simulink library "lib_all"  (Simulink 7.6)
settings: Solver=ode45  SolverMode=SingleTasking  StartTime=0.0  StopTime=10.0  RelTol=1e-3
values from neighbouring files (symbols this model uses):
  map_lib_03 = [6.8679 7.0159 7.4571 8.1849 9.1892 10.456 11.97 13.71 15.655 17.781 20.063 22.474 ... (803 elements, 73x11)]    [scr_simdata.mat]    (also in scr_simdata_all.mat)
  map_lib_06 = [2.5784 2.6727 2.9588 3.4453 4.1444 5.068 6.226 7.6242 9.2634 11.139 13.24 15.552 ... (803 elements, 73x11)]    [scr_simdata.mat]    (also in scr_simdata_all.mat)
  map_lib_09 = [0.0025784 0.0026727 0.0029588 0.0034453 0.0041444 0.005068 0.006226 0.0076242 0.0092634 0.011139 0.01324 0.015552 ... (803 elements, 73x11)]    [scr_simdata.mat]    (also in scr_simdata_all.mat)
  map_lib_10 = [2.74 2.75 2.76 2.77 2.78 2.79 2.8 2.81 2.82 2.83 2.84 2.85 ... (81 elements, 1x81)]    [scr_simdata.mat]    (also in scr_simdata_all.mat)
  map_lib_11 = [1.1983 1.2027 1.207 1.2113 1.2156 1.2198 1.2241 1.2283 1.2326 1.2368 1.241 1.2451 ... (81 elements, 1x81)]    [scr_simdata.mat]    (also in scr_simdata_all.mat)
  map_lib_12 = [2.74 2.75 2.76 2.77 2.78 2.79 2.8 2.81 2.82 2.83 2.84 2.85 ... (81 elements, 1x81)]    [scr_simdata.mat]    (also in scr_simdata_all.mat)
  map_lib_13 = [1.74 1.75 1.76 1.77 1.78 1.79 1.8 1.81 1.82 1.83 1.84 1.85 ... (81 elements, 1x81)]    [scr_simdata.mat]    (also in scr_simdata_all.mat)
  map_lib_16 = [0.00048828125 1.6159 1.8962 2.0821 2.2251 2.3426 2.4433 2.5318 2.611 2.683 2.749 2.8101 ... (1419 elements, 129x11)]    [scr_simdata.mat]    (also in scr_simdata_all.mat)
  map_mr2_01 = [0 800 1600 2400 3200 4000]    [scr_simdata.mat]    (also in scr_simdata_all.mat)
  map_mr2_02 = [1.32 1.32 1.33 1.34 1.36 1.38]    [scr_simdata.mat]    (also in scr_simdata_all.mat)
  map_mr2_03 = [0 800 1600 2400 3200 4000]    [scr_simdata.mat]    (also in scr_simdata_all.mat)
  map_mr2_04 = [1.32 1.32 1.33 1.34 1.36 1.38]    [scr_simdata.mat]    (also in scr_simdata_all.mat)
  map_mr2_05 = [0 800 1600 2400 3200 4000]    [scr_simdata.mat]    (also in scr_simdata_all.mat)
  map_mr2_06 = [1.32 1.32 1.33 1.34 1.36 1.38]    [scr_simdata.mat]    (also in scr_simdata_all.mat)
  Parameter1 = 6    [scr_simdata.mat]    (also in scr_simdata_all.mat)
  Parameter101 = 30    [scr_simdata.mat]    (also in scr_simdata_all.mat)
  Parameter102 = 12    [scr_simdata.mat]    (also in scr_simdata_all.mat)
  Parameter103 = -240    [scr_simdata.mat]    (also in scr_simdata_all.mat)
  Parameter108 = 48    [scr_simdata.mat]    (also in scr_simdata_all.mat)
  Parameter120 = 16    [scr_simdata.mat]    (also in scr_simdata_all.mat)
  Parameter121 = 87    [scr_simdata.mat]    (also in scr_simdata_all.mat)
  Parameter123 = 78    [scr_simdata.mat]    (also in scr_simdata_all.mat)
  Parameter124 = 60    [scr_simdata.mat]    (also in scr_simdata_all.mat)
  Parameter125 = -480    [scr_simdata.mat]    (also in scr_simdata_all.mat)
  Parameter130 = 96    [scr_simdata.mat]    (also in scr_simdata_all.mat)
  Parameter131 = 3    [scr_simdata.mat]    (also in scr_simdata_all.mat)
  Parameter144 = 3    [scr_simdata.mat]    (also in scr_simdata_all.mat)
  Parameter18 = 128    [scr_simdata.mat]    (also in scr_simdata_all.mat)
  Parameter19 = 16    [scr_simdata.mat]    (also in scr_simdata_all.mat)
  Parameter2 = 12    [scr_simdata.mat]    (also in scr_simdata_all.mat)
  Parameter20 = 7    [scr_simdata.mat]    (also in scr_simdata_all.mat)
  Parameter21 = 4    [scr_simdata.mat]    (also in scr_simdata_all.mat)
  Parameter3 = 18    [scr_simdata.mat]    (also in scr_simdata_all.mat)
  Parameter32 = 1    [scr_simdata.mat]    (also in scr_simdata_all.mat)
  Parameter34 = 24    [scr_simdata.mat]    (also in scr_simdata_all.mat)
  Parameter4 = 138    [scr_simdata.mat]    (also in scr_simdata_all.mat)
  Parameter42 = 48    [scr_simdata.mat]    (also in scr_simdata_all.mat)
  Parameter5 = 135    [scr_simdata.mat]    (also in scr_simdata_all.mat)
  Parameter55 = 0    [scr_simdata.mat]    (also in scr_simdata_all.mat)
  Parameter56 = 0    [scr_simdata.mat]    (also in scr_simdata_all.mat)
  Parameter57 = 1    [scr_simdata.mat]    (also in scr_simdata_all.mat)
  Parameter58 = 1    [scr_simdata.mat]    (also in scr_simdata_all.mat)
  Parameter59 = 2    [scr_simdata.mat]    (also in scr_simdata_all.mat)
  Parameter6 = 132    [scr_simdata.mat]    (also in scr_simdata_all.mat)
  Parameter60 = 2    [scr_simdata.mat]    (also in scr_simdata_all.mat)
  Parameter63 = 144    [scr_simdata.mat]    (also in scr_simdata_all.mat)
  Parameter7 = 126    [scr_simdata.mat]    (also in scr_simdata_all.mat)
  Parameter76 = 64    [scr_simdata.mat]    (also in scr_simdata_all.mat)
  Parameter77 = 135    [scr_simdata.mat]    (also in scr_simdata_all.mat)
  Parameter79 = 126    [scr_simdata.mat]    (also in scr_simdata_all.mat)
  Parameter80 = 108    [scr_simdata.mat]    (also in scr_simdata_all.mat)
  Parameter81 = 0    [scr_simdata.mat]    (also in scr_simdata_all.mat)
  Parameter86 = 0    [scr_simdata.mat]    (also in scr_simdata_all.mat)
  Parameter98 = 112    [scr_simdata.mat]    (also in scr_simdata_all.mat)
  Parameter99 = 39    [scr_simdata.mat]    (also in scr_simdata_all.mat)
undefined symbols (not defined in the file or in its folder): cylvolkp_hih_map2h, cylvolkp_map2h, pk1k_map2h
libraries: simulink x2
(part 4 of 36)

== system lib_all/SubSystem1879/SubSystem1881 ==
  note: Text370
  note: Text369
  Block5146 : Inport
  Block5147 : Inport  Port=2
  Block5148 : Constant  Value=0  OutDataTypeStr=double  SampleTime=-1
  Block5149 : Constant  Value=0  OutDataTypeStr=double  SampleTime=-1
  Block5150 : Constant  Value=720  OutDataTypeStr=double  SampleTime=-1
  Block5151 : Constant  Value=5  OutDataTypeStr=double  SampleTime=-1
  Block5152 : DataStoreRead  DataStoreName=DSM50
  Block5153 : DataStoreRead  DataStoreName=DSM35
  Block5154 : Gain  Gain=5  SaturateOnIntegerOverflow=on  OutDataTypeStr=Inherit: Same as input  ParamDataTypeStr=Inherit: Same as input
      settings: AttributesFormatString=Attribute1045
  Block5155 : Gain  Gain=5  SaturateOnIntegerOverflow=on  OutDataTypeStr=Inherit: Same as input  ParamDataTypeStr=Inherit: Same as input
      settings: AttributesFormatString=Attribute1046
  Block5156 : Gain  Gain=1/5  SaturateOnIntegerOverflow=on  OutDataTypeStr=Inherit: Same as input  ParamDataTypeStr=Inherit: Same as input
      settings: AttributesFormatString=Attribute1047
  Block5157 : Product  Inputs=*/  OutDataTypeStr=double  SaturateOnIntegerOverflow=on  RndMeth=Zero  InputSameDT=on
  Block5158 : Rounding
  Block5159 : Rounding
  Block5160 : Sum  Inputs=+-+  OutDataTypeStr=double  RndMeth=Zero  SaturateOnIntegerOverflow=on  InputSameDT=on
  Block5161 : Sum  Inputs=-+  OutDataTypeStr=double  RndMeth=Zero  SaturateOnIntegerOverflow=on  InputSameDT=on
  SubSystem1882 : Subsystem  TreatAsAtomicUnit=on  mask(***ATC.DIVISION***){bd_version=1.01.A, r0=チェック有り, r1=, r2=, r3=, r4=, r5=, r6=, r7=, r8=, r9=, c0=, c1=, c2=, c3=, c4=, c5=, c6=, c7=, c8=, c9=}
      mask prompts: bd_version "Version"
      mask help: これはAutoCからのブロックです．編集禁止．
      settings: OpenFcn=warndlg('変更禁止!');
      mask Help: web(ui_blockhelpmng(gcb));
  SubSystem1883 : Subsystem  TreatAsAtomicUnit=on  mask(***ATC.DSRVEC***){bd_version=1.01.A, r0=DSM95, r1=-1, r2=, r3=, r4=, r5=, r6=, r7=, r8=, r9=, c0=, c1=, c2=, c3=, c4=, c5=, c6=, c7=, c8=, c9=}
      mask prompts: bd_version "Version"
      mask help: これはAutoCからのブロックです．編集禁止．
      settings: OpenFcn=warndlg('変更禁止!');
      mask Help: web(ui_blockhelpmng(gcb));
      mask Display: disp('ATC.DSRVEC')
  SubSystem1884 : Subsystem
      settings: RTWSystemCode=Inline
  Block5170 : Outport
  Block5171 : Outport  Port=2
  wires:
  Block5159/1 -> Block5170/1
  Block5156/1 -> Block5159/1
  Block5155/1 -> Block5160/3
  Block5152/1 -> Block5160/2
  Block5153/1 -> SubSystem1882/2(div)
  SubSystem1883/1(Output) -> SubSystem1882/1(mult)
  Block5148/1 -> SubSystem1882/3(init)
  Block5151/1 -> Block5157/2
  Block5161/1 -> Block5171/1
  Block5147/1 -> SubSystem1883/1(element)
  Block5150/1 -> SubSystem1884/1(Block5162)
  Block5160/1 -> SubSystem1884/2(Block5163)  "SigLabel166"
  Block5149/1 -> SubSystem1884/3(Block5164)
  SubSystem1884/1(Block5169) -> Block5157/1, Block5156/1, Block5161/2
  Block5157/1 -> Block5158/1
  Block5158/1 -> Block5154/1
  Block5154/1 -> Block5161/1
  Block5146/1 -> Block5155/1
  SubSystem1882/1(Out) -> Block5160/1  "SigLabel165"

== system lib_all/SubSystem1879/SubSystem1887 ==
  note: Text373
  note: Text372
  note: Text371
  Block5172 : Inport
  Block5173 : Inport  Port=2
  Block5174 : Inport  Port=3
  Block5175 : Constant  Value=0  OutDataTypeStr=double  SampleTime=-1
  Block5176 : Constant  OutDataTypeStr=double  SampleTime=-1
  Block5177 : Constant  OutDataTypeStr=double  SampleTime=-1
  Block5178 : Constant  Value=0  OutDataTypeStr=double  SampleTime=-1
  Block5179 : Constant  OutDataTypeStr=double  SampleTime=-1
  Block5180 : Constant  Value=143  OutDataTypeStr=double  SampleTime=-1
  Block5181 : Demux  Outputs=2
  Block5182 : Logic  Operator=NOT
  Block5183 : Mux  Inputs=2
  Block5184 : Mux  Inputs=2
  Block5185 : Sum  OutDataTypeStr=double  RndMeth=Zero  SaturateOnIntegerOverflow=on  InputSameDT=on
  Block5186 : Sum  OutDataTypeStr=double  RndMeth=Zero  SaturateOnIntegerOverflow=on  InputSameDT=on
  Block5187 : Switch  Threshold=0.5  RndMeth=Zero  SaturateOnIntegerOverflow=on  InputSameDT=on
  Block5188 : Switch  Threshold=0.5  RndMeth=Zero  SaturateOnIntegerOverflow=on  InputSameDT=on
  Block5189 : Switch  Threshold=0.5  RndMeth=Zero  SaturateOnIntegerOverflow=on  InputSameDT=on
  SubSystem1888 : Subsystem
  SubSystem1889 : Subsystem
  SubSystem1890 : Subsystem
  SubSystem1891 : Subsystem  TreatAsAtomicUnit=on
  SubSystem1900 : Subsystem  TreatAsAtomicUnit=on
  SubSystem1910 : Subsystem  TreatAsAtomicUnit=on
  Block5234 : Outport
  Block5235 : Outport  Port=2
  wires:
  Block5172/1 -> SubSystem1888/1(In1), Block5185/2, Block5183/1
  Block5180/1 -> SubSystem1888/2(In2), Block5184/1
  Block5173/1 -> SubSystem1889/1(In1), SubSystem1890/1(In1)
  Block5175/1 -> SubSystem1889/2(In2)
  SubSystem1889/1(Out1) -> Block5187/2, SubSystem1891/Enable
  SubSystem1891/1(Block5203) -> Block5187/1
  Block5176/1 -> SubSystem1890/2(In2)
  SubSystem1890/1(Out1) -> Block5188/2, SubSystem1900/Enable, Block5182/1
  SubSystem1900/1(Block5218) -> Block5188/1
  Block5182/1 -> SubSystem1910/Enable
  Block5188/1 -> Block5187/3
  SubSystem1910/1(Block5233) -> Block5188/3
  Block5181/1 -> Block5234/1
  Block5181/2 -> Block5235/1
  Block5187/1 -> Block5181/1
  SubSystem1888/1(Out1) -> Block5189/2
  Block5177/1 -> Block5185/1
  Block5178/1 -> Block5184/2
  Block5184/1 -> Block5189/3
  Block5185/1 -> Block5183/2
  Block5183/1 -> Block5189/1
  Block5174/1 -> SubSystem1891/2(Block5191), SubSystem1900/2(Block5205), SubSystem1910/2(Block5220)
  Block5189/1 -> Block5186/2
  Block5179/1 -> Block5186/1
  Block5186/1 -> SubSystem1891/1(Block5190), SubSystem1900/1(Block5204), SubSystem1910/1(Block5219)

== system lib_all/SubSystem1920/SubSystem1922 ==
  note: Text378
  note: Text377
  Block5246 : Inport
  Block5247 : Inport  Port=2
  Block5248 : Constant  Value=0  OutDataTypeStr=double  SampleTime=-1
  Block5249 : Constant  Value=0  OutDataTypeStr=double  SampleTime=-1
  Block5250 : Constant  Value=720  OutDataTypeStr=double  SampleTime=-1
  Block5251 : Constant  Value=5  OutDataTypeStr=double  SampleTime=-1
  Block5252 : DataStoreRead  DataStoreName=DSM50
  Block5253 : DataStoreRead  DataStoreName=DSM35
  Block5254 : Gain  Gain=5  SaturateOnIntegerOverflow=on  OutDataTypeStr=Inherit: Same as input  ParamDataTypeStr=Inherit: Same as input
      settings: AttributesFormatString=Attribute1061
  Block5255 : Gain  Gain=5  SaturateOnIntegerOverflow=on  OutDataTypeStr=Inherit: Same as input  ParamDataTypeStr=Inherit: Same as input
      settings: AttributesFormatString=Attribute1062
  Block5256 : Gain  Gain=1/5  SaturateOnIntegerOverflow=on  OutDataTypeStr=Inherit: Same as input  ParamDataTypeStr=Inherit: Same as input
      settings: AttributesFormatString=Attribute1063
  Block5257 : Product  Inputs=*/  OutDataTypeStr=double  SaturateOnIntegerOverflow=on  RndMeth=Zero  InputSameDT=on
  Block5258 : Rounding
  Block5259 : Rounding
  Block5260 : Sum  Inputs=+-+  OutDataTypeStr=double  RndMeth=Zero  SaturateOnIntegerOverflow=on  InputSameDT=on
  Block5261 : Sum  Inputs=-+  OutDataTypeStr=double  RndMeth=Zero  SaturateOnIntegerOverflow=on  InputSameDT=on
  SubSystem1923 : Subsystem  TreatAsAtomicUnit=on  mask(***ATC.DIVISION***){bd_version=1.01.A, r0=チェック有り, r1=, r2=, r3=, r4=, r5=, r6=, r7=, r8=, r9=, c0=, c1=, c2=, c3=, c4=, c5=, c6=, c7=, c8=, c9=}
      mask prompts: bd_version "Version"
      mask help: これはAutoCからのブロックです．編集禁止．
      settings: OpenFcn=warndlg('変更禁止!');
      mask Help: web(ui_blockhelpmng(gcb));
  SubSystem1924 : Subsystem  TreatAsAtomicUnit=on  mask(***ATC.DSRVEC***){bd_version=1.01.A, r0=DSM95, r1=-1, r2=, r3=, r4=, r5=, r6=, r7=, r8=, r9=, c0=, c1=, c2=, c3=, c4=, c5=, c6=, c7=, c8=, c9=}
      mask prompts: bd_version "Version"
      mask help: これはAutoCからのブロックです．編集禁止．
      settings: OpenFcn=warndlg('変更禁止!');
      mask Help: web(ui_blockhelpmng(gcb));
      mask Display: disp('ATC.DSRVEC')
  SubSystem1925 : Subsystem
      settings: RTWSystemCode=Inline
  Block5270 : Outport
  Block5271 : Outport  Port=2
  wires:
  SubSystem1923/1(Out) -> Block5260/1  "SigLabel169"
  Block5246/1 -> Block5255/1
  Block5254/1 -> Block5261/1
  Block5258/1 -> Block5254/1
  Block5257/1 -> Block5258/1
  SubSystem1925/1(Block5269) -> Block5261/2, Block5256/1, Block5257/1
  Block5249/1 -> SubSystem1925/3(Block5264)
  Block5260/1 -> SubSystem1925/2(Block5263)  "SigLabel168"
  Block5250/1 -> SubSystem1925/1(Block5262)
  Block5247/1 -> SubSystem1924/1(element)
  Block5261/1 -> Block5271/1
  Block5251/1 -> Block5257/2
  Block5248/1 -> SubSystem1923/3(init)
  SubSystem1924/1(Output) -> SubSystem1923/1(mult)
  Block5253/1 -> SubSystem1923/2(div)
  Block5252/1 -> Block5260/2
  Block5255/1 -> Block5260/3
  Block5256/1 -> Block5259/1
  Block5259/1 -> Block5270/1

== system lib_all/SubSystem1920/SubSystem1928 ==
  note: Text381
  note: Text380
  note: Text379
  Block5272 : Inport
  Block5273 : Inport  Port=2
  Block5274 : Inport  Port=3
  Block5275 : Constant  Value=0  OutDataTypeStr=double  SampleTime=-1
  Block5276 : Constant  OutDataTypeStr=double  SampleTime=-1
  Block5277 : Constant  OutDataTypeStr=double  SampleTime=-1
  Block5278 : Constant  Value=0  OutDataTypeStr=double  SampleTime=-1
  Block5279 : Constant  OutDataTypeStr=double  SampleTime=-1
  Block5280 : Constant  Value=143  OutDataTypeStr=double  SampleTime=-1
  Block5281 : Demux  Outputs=2
  Block5282 : Logic  Operator=NOT
  Block5283 : Mux  Inputs=2
  Block5284 : Mux  Inputs=2
  Block5285 : Sum  OutDataTypeStr=double  RndMeth=Zero  SaturateOnIntegerOverflow=on  InputSameDT=on
  Block5286 : Sum  OutDataTypeStr=double  RndMeth=Zero  SaturateOnIntegerOverflow=on  InputSameDT=on
  Block5287 : Switch  Threshold=0.5  RndMeth=Zero  SaturateOnIntegerOverflow=on  InputSameDT=on
  Block5288 : Switch  Threshold=0.5  RndMeth=Zero  SaturateOnIntegerOverflow=on  InputSameDT=on
  Block5289 : Switch  Threshold=0.5  RndMeth=Zero  SaturateOnIntegerOverflow=on  InputSameDT=on
  SubSystem1929 : Subsystem
  SubSystem1930 : Subsystem
  SubSystem1931 : Subsystem
  SubSystem1932 : Subsystem  TreatAsAtomicUnit=on
  SubSystem1941 : Subsystem  TreatAsAtomicUnit=on
  SubSystem1951 : Subsystem  TreatAsAtomicUnit=on
  Block5334 : Outport
  Block5335 : Outport  Port=2
  wires:
  Block5286/1 -> SubSystem1951/1(Block5319), SubSystem1941/1(Block5304), SubSystem1932/1(Block5290)
  Block5279/1 -> Block5286/1
  Block5289/1 -> Block5286/2
  Block5274/1 -> SubSystem1951/2(Block5320), SubSystem1941/2(Block5305), SubSystem1932/2(Block5291)
  Block5283/1 -> Block5289/1
  Block5285/1 -> Block5283/2
  Block5284/1 -> Block5289/3
  Block5278/1 -> Block5284/2
  Block5277/1 -> Block5285/1
  SubSystem1929/1(Out1) -> Block5289/2
  Block5287/1 -> Block5281/1
  Block5281/2 -> Block5335/1
  Block5281/1 -> Block5334/1
  SubSystem1951/1(Block5333) -> Block5288/3
  Block5288/1 -> Block5287/3
  Block5282/1 -> SubSystem1951/Enable
  SubSystem1941/1(Block5318) -> Block5288/1
  SubSystem1931/1(Out1) -> Block5282/1, SubSystem1941/Enable, Block5288/2
  Block5276/1 -> SubSystem1931/2(In2)
  SubSystem1932/1(Block5303) -> Block5287/1
  SubSystem1930/1(Out1) -> SubSystem1932/Enable, Block5287/2
  Block5275/1 -> SubSystem1930/2(In2)
  Block5273/1 -> SubSystem1931/1(In1), SubSystem1930/1(In1)
  Block5280/1 -> Block5284/1, SubSystem1929/2(In2)
  Block5272/1 -> Block5283/1, Block5285/2, SubSystem1929/1(In1)

== system lib_all/SubSystem3/SubSystem4 ==
  note: ***編集禁止***
  element : Inport
  Inside_DSR : DataStoreRead  DataStoreName=DSM10
  Selector : Selector  IndexOptions=Index vector (port)  Indices=[1 3]  OutputSizes=1
  Output : Outport
  wires:
  Selector/1 -> Output/1
  element/1 -> Selector/2
  Inside_DSR/1 -> Selector/1

== system lib_all/SubSystem3/SubSystem5 ==
  note: ***編集禁止***
  element : Inport
  Inside_DSR : DataStoreRead  DataStoreName=DSM16
  Selector : Selector  IndexOptions=Index vector (port)  Indices=[1 3]  OutputSizes=1
  Output : Outport
  wires:
  Inside_DSR/1 -> Selector/1
  element/1 -> Selector/2
  Selector/1 -> Output/1

== system lib_all/SubSystem3/SubSystem6 ==
  note: ***編集禁止***
  element : Inport
  Inside_DSR : DataStoreRead  DataStoreName=DSM17
  Selector : Selector  IndexOptions=Index vector (port)  Indices=[1 3]  OutputSizes=1
  Output : Outport
  wires:
  Selector/1 -> Output/1
  element/1 -> Selector/2
  Inside_DSR/1 -> Selector/1

== system lib_all/SubSystem347/SubSystem349 ==
  Block762 : Inport
  Block763 : Constant  OutDataTypeStr=double  SampleTime=-1
  Block764 : Constant  Value=Parameter18  OutDataTypeStr=double  SampleTime=-1
  Block765 : Demux  Outputs=2
  Block766 : Sum  OutDataTypeStr=double  RndMeth=Zero  SaturateOnIntegerOverflow=on  InputSameDT=on
  SubSystem350 : Subsystem
  SubSystem351 : Subsystem  TreatAsAtomicUnit=on  mask(***ATC.DSRVEC***){bd_version=1.01.A, r0=DSM386, r1=-1, r2=, r3=, r4=, r5=, r6=, r7=, r8=, r9=, c0=, c1=, c2=, c3=, c4=, c5=, c6=, c7=, c8=, c9=}
      mask prompts: bd_version "Version"
      mask help: これはAutoCからのブロックです．編集禁止．
      settings: OpenFcn=warndlg('変更禁止!');
      mask Help: web(ui_blockhelpmng(gcb));
      mask Display: disp('ATC.DSRVEC')
  SubSystem352 : Subsystem
  SubSystem476 : Subsystem  TreatAsAtomicUnit=on
      settings: RTWFcnNameOpts=Use subsystem name
  wires:
  Block766/1 -> SubSystem351/1(element), SubSystem476/1(Block1053)
  SubSystem476/1(Block1064) -> Block765/1
  Block763/1 -> Block766/2
  Block765/1 -> SubSystem352/2(Block768)  "SigLabel24"
  Block765/2 -> SubSystem352/3(Block769)  "SigLabel23"
  Block762/1 -> Block766/1, SubSystem352/1(Block767)
  SubSystem351/1(Output) -> SubSystem350/1(In1), SubSystem352/4(Block770)
  Block764/1 -> SubSystem350/2(In2)
  SubSystem350/1(Out1) -> SubSystem352/Enable

== system lib_all/SubSystem347/SubSystem477 ==
  note: Text69
  Block1065 : Inport
  Block1066 : Constant  Value=Parameter18  OutDataTypeStr=double  SampleTime=-1
  Block1067 : Constant  OutDataTypeStr=double  SampleTime=-1
  Block1068 : Sum  Inputs=|++  OutDataTypeStr=double  RndMeth=Zero  SaturateOnIntegerOverflow=on  InputSameDT=on
  SubSystem478 : Subsystem
  SubSystem479 : Subsystem
  SubSystem502 : Subsystem  TreatAsAtomicUnit=on  mask(***ATC.DSRVEC***){bd_version=1.01.A, r0=DSM386, r1=-1, r2=, r3=, r4=, r5=, r6=, r7=, r8=, r9=, c0=, c1=, c2=, c3=, c4=, c5=, c6=, c7=, c8=, c9=}
      mask prompts: bd_version "Version"
      mask help: これはAutoCからのブロックです．編集禁止．
      settings: OpenFcn=warndlg('変更禁止!');
      mask Help: web(ui_blockhelpmng(gcb));
      mask Display: disp('ATC.DSRVEC')
  wires:
  Block1067/1 -> Block1068/2
  Block1068/1 -> SubSystem502/1(element), SubSystem479/1(Block1069)
  SubSystem502/1(Output) -> SubSystem478/1(In1)
  Block1065/1 -> Block1068/1
  Block1066/1 -> SubSystem478/2(In2)
  SubSystem478/1(Out1) -> SubSystem479/Enable

== system lib_all/SubSystem347/SubSystem503 ==
  Block1115 : Constant  Value=Parameter18  OutDataTypeStr=double  SampleTime=-1
  Block1116 : Constant  Value=Parameter131  OutDataTypeStr=double  SampleTime=-1
  Block1117 : DataStoreRead  DataStoreName=DSM386
  Block1118 : Sum  Inputs=+  OutDataTypeStr=double  RndMeth=Zero  SaturateOnIntegerOverflow=on  InputSameDT=on
  SubSystem504 : Subsystem
  SubSystem505 : Subsystem
  SubSystem506 : Subsystem  TreatAsAtomicUnit=on
  wires:
  Block1117/1 -> SubSystem504/1(In1)
  Block1115/1 -> SubSystem504/2(In2)
  SubSystem504/1(Out1) -> Block1118/1
  Block1118/1 -> SubSystem505/1(In1)
  Block1116/1 -> SubSystem505/2(In2)
  SubSystem505/1(Out1) -> SubSystem506/Enable

== system lib_all/SubSystem518/SubSystem520 ==
  Block1137 : Inport
  Block1138 : Constant  OutDataTypeStr=double  SampleTime=-1
  Block1139 : Demux  Outputs=2
  Block1140 : Sum  OutDataTypeStr=double  RndMeth=Zero  SaturateOnIntegerOverflow=on  InputSameDT=on
  Block1141 : Terminator
  SubSystem521 : Subsystem
      settings: RTWSystemCode=Function  RTWFcnNameOpts=Use subsystem name
  SubSystem522 : Subsystem  TreatAsAtomicUnit=on  mask(***ATC.DSRVEC***){bd_version=1.01.A, r0=DSM36, r1=-1, r2=, r3=, r4=, r5=, r6=, r7=, r8=, r9=, c0=, c1=, c2=, c3=, c4=, c5=, c6=, c7=, c8=, c9=}
      mask prompts: bd_version "Version"
      mask help: これはAutoCからのブロックです．編集禁止．
      settings: OpenFcn=warndlg('変更禁止!');
      mask Help: web(ui_blockhelpmng(gcb));
      mask Display: disp('ATC.DSRVEC')
  Block1154 : Outport
  Block1155 : Outport  Port=2
  wires:
  SubSystem521/1(Block1153) -> Block1139/1
  Block1140/1 -> SubSystem522/1(element), SubSystem521/1(Block1142)
  Block1137/1 -> Block1140/1
  Block1139/2 -> Block1155/1
  Block1139/1 -> Block1154/1
  Block1138/1 -> Block1140/2
  SubSystem522/1(Output) -> Block1141/1

== system lib_all/SubSystem518/SubSystem523 ==
  Block1156 : Inport
  Block1157 : Inport  Port=2
  Block1158 : Inport  Port=3
  Block1159 : Constant  Value=0  OutDataTypeStr=double  SampleTime=-1
  Block1160 : Constant  Value=3  OutDataTypeStr=double  SampleTime=-1
  Block1161 : Constant  Value=3  OutDataTypeStr=double  SampleTime=-1
  Block1162 : Constant  Value=0  OutDataTypeStr=double  SampleTime=-1
  SubSystem524 : Subsystem  TreatAsAtomicUnit=on
      settings: RTWFcnNameOpts=Use subsystem name
  SubSystem577 : Subsystem  TreatAsAtomicUnit=on
      settings: RTWFcnNameOpts=Use subsystem name
  Block1407 : Outport
  Block1408 : Outport  Port=2
  wires:
  Block1156/1 -> SubSystem524/1(Block1163), SubSystem577/1(Block1285)
  Block1157/1 -> SubSystem524/2(Block1164)
  Block1158/1 -> SubSystem577/2(Block1286)
  Block1162/1 -> SubSystem524/3(Block1165)
  Block1159/1 -> SubSystem577/3(Block1287)
  Block1160/1 -> SubSystem524/4(Block1166)
  Block1161/1 -> SubSystem577/4(Block1288)
  SubSystem524/1(Block1284) -> Block1407/1  "SigLabel33"
  SubSystem577/1(Block1406) -> Block1408/1  "SigLabel32"

== system lib_all/SubSystem518/SubSystem630 ==
  note: Text89
  Block1409 : Inport
  Block1410 : Inport  Port=2
  Block1411 : Inport  Port=3
  Block1412 : Constant  OutDataTypeStr=double  SampleTime=-1
  Block1413 : Constant  Value=2048/64/256  OutDataTypeStr=double  SampleTime=-1
  Block1414 : DataStoreRead  DataStoreName=DSM15
  Block1415 : DataStoreRead  DataStoreName=DSM15
  Block1422 : Sum  Inputs=|++  OutDataTypeStr=double  RndMeth=Zero  SaturateOnIntegerOverflow=on  InputSameDT=on
  Block1423 : Switch  Threshold=0.5  RndMeth=Zero  SaturateOnIntegerOverflow=on  InputSameDT=on
  Block1424 : Lookup  InputValues=map_lib_10  Table=map_lib_11  SaturateOnIntegerOverflow=on
      settings: AttributesFormatString=<%<OutputValues>>\nAttribute295
  Block1425 : Lookup  InputValues=map_lib_12  Table=map_lib_13  SaturateOnIntegerOverflow=on
      settings: AttributesFormatString=<%<OutputValues>>\nAttribute296
  SubSystem631 : Subsystem  TreatAsAtomicUnit=on  mask(***ATC.DIVISION***){bd_version=1.01.A, r0=チェック無し, r1=, r2=, r3=, r4=, r5=, r6=, r7=, r8=, r9=, c0=, c1=, c2=, c3=, c4=, c5=, c6=, c7=, c8=, c9=}
      mask prompts: bd_version "Version"
      mask help: これはAutoCからのブロックです．編集禁止．
      settings: OpenFcn=warndlg('変更禁止!');
      mask Help: web(ui_blockhelpmng(gcb));
  SubSystem632 : Subsystem
  SubSystem633 : Subsystem  TreatAsAtomicUnit=on  mask(***ATC.DSWVEC***){bd_version=1.01.A, r0=DSM45, r1=-1, r2=, r3=, r4=, r5=, r6=, r7=, r8=, r9=, c0=, c1=, c2=, c3=, c4=, c5=, c6=, c7=, c8=, c9=}
      mask prompts: bd_version "Version"
      mask help: これはAutoCからのブロックです．編集禁止．
      settings: OpenFcn=warndlg('変更禁止!');
      mask Help: web(ui_blockhelpmng(gcb));
      mask Display: disp('ATC.DSRVEC')
  SubSystem634 : Subsystem  TreatAsAtomicUnit=on  mask(***ATC.DSWVEC***){bd_version=1.01.A, r0=DSM46, r1=-1, r2=, r3=, r4=, r5=, r6=, r7=, r8=, r9=, c0=, c1=, c2=, c3=, c4=, c5=, c6=, c7=, c8=, c9=}
      mask prompts: bd_version "Version"
      mask help: これはAutoCからのブロックです．編集禁止．
      settings: OpenFcn=warndlg('変更禁止!');
      mask Help: web(ui_blockhelpmng(gcb));
      mask Display: disp('ATC.DSRVEC')
  SubSystem635 : Subsystem  TreatAsAtomicUnit=on  mask(***ATC.DSWVEC***){bd_version=1.01.A, r0=DSM82, r1=-1, r2=, r3=, r4=, r5=, r6=, r7=, r8=, r9=, c0=, c1=, c2=, c3=, c4=, c5=, c6=, c7=, c8=, c9=}
      mask prompts: bd_version "Version"
      mask help: これはAutoCからのブロックです．編集禁止．
      settings: OpenFcn=warndlg('変更禁止!');
      mask Help: web(ui_blockhelpmng(gcb));
      mask Display: disp('ATC.DSRVEC')
  SubSystem636 : Subsystem  TreatAsAtomicUnit=on  mask(***ATC.DSWVEC***){bd_version=1.01.A, r0=DSM83, r1=-1, r2=, r3=, r4=, r5=, r6=, r7=, r8=, r9=, c0=, c1=, c2=, c3=, c4=, c5=, c6=, c7=, c8=, c9=}
      mask prompts: bd_version "Version"
      mask help: これはAutoCからのブロックです．編集禁止．
      settings: OpenFcn=warndlg('変更禁止!');
      mask Help: web(ui_blockhelpmng(gcb));
      mask Display: disp('ATC.DSRVEC')
  SubSystem637 : Subsystem  TreatAsAtomicUnit=on  mask(***ATC.DSRVEC***){bd_version=1.01.A, r0=DSM84, r1=-1, r2=, r3=, r4=, r5=, r6=, r7=, r8=, r9=, c0=, c1=, c2=, c3=, c4=, c5=, c6=, c7=, c8=, c9=}
      mask prompts: bd_version "Version"
      mask help: これはAutoCからのブロックです．編集禁止．
      settings: OpenFcn=warndlg('変更禁止!');
      mask Help: web(ui_blockhelpmng(gcb));
      mask Display: disp('ATC.DSRVEC')
  SubSystem638 : Subsystem  TreatAsAtomicUnit=on  mask(***ATC.DSWVEC***){bd_version=1.01.A, r0=DSM84, r1=-1, r2=, r3=, r4=, r5=, r6=, r7=, r8=, r9=, c0=, c1=, c2=, c3=, c4=, c5=, c6=, c7=, c8=, c9=}
      mask prompts: bd_version "Version"
      mask help: これはAutoCからのブロックです．編集禁止．
      settings: OpenFcn=warndlg('変更禁止!');
      mask Help: web(ui_blockhelpmng(gcb));
      mask Display: disp('ATC.DSRVEC')
  SubSystem639 : Subsystem  TreatAsAtomicUnit=on  mask(***ATC.DSRVEC***){bd_version=1.01.A, r0=DSM9, r1=-1, r2=, r3=, r4=, r5=, r6=, r7=, r8=, r9=, c0=, c1=, c2=, c3=, c4=, c5=, c6=, c7=, c8=, c9=}
      mask prompts: bd_version "Version"
      mask help: これはAutoCからのブロックです．編集禁止．
      settings: OpenFcn=warndlg('変更禁止!');
      mask Help: web(ui_blockhelpmng(gcb));
      mask Display: disp('ATC.DSRVEC')
  SubSystem640 : Subsystem  TreatAsAtomicUnit=on  mask(***ATC.DSWVEC***){bd_version=1.01.A, r0=DSM9, r1=-1, r2=, r3=, r4=, r5=, r6=, r7=, r8=, r9=, c0=, c1=, c2=, c3=, c4=, c5=, c6=, c7=, c8=, c9=}
      mask prompts: bd_version "Version"
      mask help: これはAutoCからのブロックです．編集禁止．
      settings: OpenFcn=warndlg('変更禁止!');
      mask Help: web(ui_blockhelpmng(gcb));
      mask Display: disp('ATC.DSRVEC')
  SubSystem641 : Subsystem  TreatAsAtomicUnit=on
  SubSystem642 : Subsystem  TreatAsAtomicUnit=on
  wires:
  Block1423/1 -> SubSystem631/2(div)
  Block1413/1 -> SubSystem632/2(In2), Block1423/1
  SubSystem632/1(Out1) -> Block1423/2
  Block1412/1 -> Block1422/2
  Block1409/1 -> Block1422/1
  SubSystem631/1(Out) -> Block1424/1, Block1425/1  "SigLabel36"
  Block1424/1 -> SubSystem641/2(Block1427), SubSystem635/1(input)
  SubSystem639/1(Output) -> SubSystem641/1(Block1426)
  SubSystem641/1(Block1434) -> SubSystem640/1(input)
  Block1415/1 -> SubSystem641/3(Block1428)
  Block1425/1 -> SubSystem642/2(Block1436), SubSystem636/1(input)
  SubSystem637/1(Output) -> SubSystem642/1(Block1435)
  Block1414/1 -> SubSystem642/3(Block1437)
  SubSystem642/1(Block1443) -> SubSystem638/1(input)
  Block1411/1 -> Block1423/3, SubSystem632/1(In1), [TagName8]
  Block1410/1 -> SubSystem631/1(mult), [TagName7]
  [TagName7] -> SubSystem633/1(input)
  [TagName8] -> SubSystem634/1(input)
  [TagName5] -> SubSystem633/2(element), SubSystem634/2(element)
  Block1422/1 -> SubSystem635/2(element), SubSystem640/2(element), SubSystem639/1(element), SubSystem637/1(element), SubSystem638/2(element), SubSystem636/2(element), [TagName5]

== system lib_all/SubSystem643/SubSystem645 ==
  Block1444 : DataStoreRead  DataStoreName=DSM40
      settings: AttributesFormatString=Attribute301
  Block1445 : DataStoreRead  DataStoreName=DSM54
      settings: AttributesFormatString=Attribute302
  Block1446 : DataStoreWrite  DataStoreName=DSM78
      settings: AttributesFormatString=Attribute303
  Block1447 : MultiPortSwitch  Inputs=10  zeroidx=off  RndMeth=Zero  SaturateOnIntegerOverflow=on  InputSameDT=on
  SubSystem646 : Subsystem  TreatAsAtomicUnit=on  mask(***ATC.DSRVEC***){bd_version=1.01.A, r0=DSM77, r1=-1, r2=, r3=, r4=, r5=, r6=, r7=, r8=, r9=, c0=, c1=, c2=, c3=, c4=, c5=, c6=, c7=, c8=, c9=}
      mask prompts: bd_version "Version"
      mask help: これはAutoCからのブロックです．編集禁止．
      settings: OpenFcn=warndlg('変更禁止!');
      mask Help: web(ui_blockhelpmng(gcb));
      mask Display: disp('ATC.DSRVEC')
  SubSystem647 : Subsystem  TreatAsAtomicUnit=on  mask(***ATC.DSRVEC***){bd_version=1.01.A, r0=DSM39, r1=-1, r2=, r3=, r4=, r5=, r6=, r7=, r8=, r9=, c0=, c1=, c2=, c3=, c4=, c5=, c6=, c7=, c8=, c9=}
      mask prompts: bd_version "Version"
      mask help: これはAutoCからのブロックです．編集禁止．
      settings: OpenFcn=warndlg('変更禁止!');
      mask Help: web(ui_blockhelpmng(gcb));
      mask Display: disp('ATC.DSRVEC')
  SubSystem648 : Subsystem  TreatAsAtomicUnit=on  mask(***ATC.DSRVEC***){bd_version=1.01.A, r0=DSM51, r1=-1, r2=, r3=, r4=, r5=, r6=, r7=, r8=, r9=, c0=, c1=, c2=, c3=, c4=, c5=, c6=, c7=, c8=, c9=}
      mask prompts: bd_version "Version"
      mask help: これはAutoCからのブロックです．編集禁止．
      settings: OpenFcn=warndlg('変更禁止!');
      mask Help: web(ui_blockhelpmng(gcb));
      mask Display: disp('ATC.DSRVEC')
  SubSystem649 : Subsystem  TreatAsAtomicUnit=on  mask(***ATC.DSRVEC***){bd_version=1.01.A, r0=DSM72, r1=-1, r2=, r3=, r4=, r5=, r6=, r7=, r8=, r9=, c0=, c1=, c2=, c3=, c4=, c5=, c6=, c7=, c8=, c9=}
      mask prompts: bd_version "Version"
      mask help: これはAutoCからのブロックです．編集禁止．
      settings: OpenFcn=warndlg('変更禁止!');
      mask Help: web(ui_blockhelpmng(gcb));
      mask Display: disp('ATC.DSRVEC')
  SubSystem650 : Subsystem  TreatAsAtomicUnit=on  mask(***ATC.DSRVEC***){bd_version=1.01.A, r0=DSM53, r1=-1, r2=, r3=, r4=, r5=, r6=, r7=, r8=, r9=, c0=, c1=, c2=, c3=, c4=, c5=, c6=, c7=, c8=, c9=}
      mask prompts: bd_version "Version"
      mask help: これはAutoCからのブロックです．編集禁止．
      settings: OpenFcn=warndlg('変更禁止!');
      mask Help: web(ui_blockhelpmng(gcb));
      mask Display: disp('ATC.DSRVEC')
  SubSystem651 : Subsystem  TreatAsAtomicUnit=on  mask(***ATC.DSRVEC***){bd_version=1.01.A, r0=DSM86, r1=-1, r2=, r3=, r4=, r5=, r6=, r7=, r8=, r9=, c0=, c1=, c2=, c3=, c4=, c5=, c6=, c7=, c8=, c9=}
      mask prompts: bd_version "Version"
      mask help: これはAutoCからのブロックです．編集禁止．
      settings: OpenFcn=warndlg('変更禁止!');
      mask Help: web(ui_blockhelpmng(gcb));
      mask Display: disp('ATC.DSRVEC')
  SubSystem652 : Subsystem  TreatAsAtomicUnit=on  mask(***ATC.DSRVEC***){bd_version=1.01.A, r0=DSM62, r1=-1, r2=, r3=, r4=, r5=, r6=, r7=, r8=, r9=, c0=, c1=, c2=, c3=, c4=, c5=, c6=, c7=, c8=, c9=}
      mask prompts: bd_version "Version"
      mask help: これはAutoCからのブロックです．編集禁止．
      settings: OpenFcn=warndlg('変更禁止!');
      mask Help: web(ui_blockhelpmng(gcb));
      mask Display: disp('ATC.DSRVEC')
  SubSystem653 : Subsystem  TreatAsAtomicUnit=on  mask(***ATC.DSRVEC***){bd_version=1.01.A, r0=DSM79, r1=-1, r2=, r3=, r4=, r5=, r6=, r7=, r8=, r9=, c0=, c1=, c2=, c3=, c4=, c5=, c6=, c7=, c8=, c9=}
      mask prompts: bd_version "Version"
      mask help: これはAutoCからのブロックです．編集禁止．
      settings: OpenFcn=warndlg('変更禁止!');
      mask Help: web(ui_blockhelpmng(gcb));
      mask Display: disp('ATC.DSRVEC')
  SubSystem654 : Subsystem  TreatAsAtomicUnit=on  mask(***ATC.DSRVEC***){bd_version=1.01.A, r0=DSM81, r1=-1, r2=, r3=, r4=, r5=, r6=, r7=, r8=, r9=, c0=, c1=, c2=, c3=, c4=, c5=, c6=, c7=, c8=, c9=}
      mask prompts: bd_version "Version"
      mask help: これはAutoCからのブロックです．編集禁止．
      settings: OpenFcn=warndlg('変更禁止!');
      mask Help: web(ui_blockhelpmng(gcb));
      mask Display: disp('ATC.DSRVEC')
  SubSystem655 : Subsystem  TreatAsAtomicUnit=on  mask(***ATC.DSRVEC***){bd_version=1.01.A, r0=DSM80, r1=-1, r2=, r3=, r4=, r5=, r6=, r7=, r8=, r9=, c0=, c1=, c2=, c3=, c4=, c5=, c6=, c7=, c8=, c9=}
      mask prompts: bd_version "Version"
      mask help: これはAutoCからのブロックです．編集禁止．
      settings: OpenFcn=warndlg('変更禁止!');
      mask Help: web(ui_blockhelpmng(gcb));
      mask Display: disp('ATC.DSRVEC')
  SubSystem656 : Subsystem  TreatAsAtomicUnit=on  mask(***ATC.SIMSTART***){bd_version=1.01.A, r0=None, r1=connect, r2=, r3=, r4=, r5=, r6=, r7=, r8=, r9=, c0=, c1=, c2=, c3=, c4=, c5=, c6=, c7=, c8=, c9=}
      mask prompts: bd_version "Version"
      mask help: ***編集禁止***connect
      settings: AttributesFormatString=Attribute304  OpenFcn=warndlg('変更禁止!');
      mask Help: web(ui_blockhelpmng(gcb));
      mask Display: disp('Start')
  wires:
  Block1447/1 -> Block1446/1
  SubSystem655/1(Output) -> Block1447/11
  SubSystem654/1(Output) -> Block1447/10
  SubSystem653/1(Output) -> Block1447/9
  SubSystem652/1(Output) -> Block1447/8
  SubSystem651/1(Output) -> Block1447/7
  SubSystem650/1(Output) -> Block1447/6
  SubSystem649/1(Output) -> Block1447/5
  SubSystem648/1(Output) -> Block1447/4
  SubSystem647/1(Output) -> Block1447/3
  SubSystem646/1(Output) -> Block1447/2
  Block1444/1 -> SubSystem650/1(element), SubSystem651/1(element), SubSystem652/1(element), SubSystem653/1(element), SubSystem654/1(element), SubSystem655/1(element), SubSystem649/1(element), SubSystem648/1(element), SubSystem647/1(element), SubSystem646/1(element)
  Block1445/1 -> Block1447/1

== system lib_all/SubSystem657/SubSystem658 ==
  note: ***編集禁止***
  mult : Inport
  div : Inport  Port=2
  Product : Product  Inputs=*/  SaturateOnIntegerOverflow=on  RndMeth=Zero  InputSameDT=on  OutDataTypeStr=Inherit: Same as first input
  Out : Outport
  wires:
  Product/1 -> Out/1
  mult/1 -> Product/1
  div/1 -> Product/2

== system lib_all/SubSystem657/SubSystem659 ==
  note: ***編集禁止***
  Input : Inport
  Min : Inport  Port=2
  Max1 : MinMax  Function=max  Inputs=2  SaturateOnIntegerOverflow=on  InputSameDT=on
  Output : Outport
  wires:
  Max1/1 -> Output/1
  Input/1 -> Max1/1
  Min/1 -> Max1/2

== system lib_all/SubSystem660/SubSystem662 ==
  note: ***編集禁止***
  In1 : Inport
  In2 : Inport  Port=2
  RO1 : RelationalOperator  Operator===  InputSameDT=on
  single1 : DataTypeConversion  OutDataTypeStr=single  SaturateOnIntegerOverflow=on  RndMeth=Zero
  single2 : DataTypeConversion  OutDataTypeStr=single  SaturateOnIntegerOverflow=on  RndMeth=Zero
  Out1 : Outport
  wires:
  RO1/1 -> Out1/1
  In2/1 -> single2/1
  In1/1 -> single1/1
  single1/1 -> RO1/1
  single2/1 -> RO1/2

== system lib_all/SubSystem660/SubSystem663 ==
  note: ***編集禁止***
  In1 : Inport
  In2 : Inport  Port=2
  RO1 : RelationalOperator  Operator===  InputSameDT=on
  single1 : DataTypeConversion  OutDataTypeStr=single  SaturateOnIntegerOverflow=on  RndMeth=Zero
  single2 : DataTypeConversion  OutDataTypeStr=single  SaturateOnIntegerOverflow=on  RndMeth=Zero
  Out1 : Outport
  wires:
  RO1/1 -> Out1/1
  In2/1 -> single2/1
  In1/1 -> single1/1
  single1/1 -> RO1/1
  single2/1 -> RO1/2

== system lib_all/SubSystem660/SubSystem664 ==
  note: ***編集禁止***
  In1 : Inport
  In2 : Inport  Port=2
  RO1 : RelationalOperator  Operator===  InputSameDT=on
  single1 : DataTypeConversion  OutDataTypeStr=single  SaturateOnIntegerOverflow=on  RndMeth=Zero
  single2 : DataTypeConversion  OutDataTypeStr=single  SaturateOnIntegerOverflow=on  RndMeth=Zero
  Out1 : Outport
  wires:
  RO1/1 -> Out1/1
  In2/1 -> single2/1
  In1/1 -> single1/1
  single1/1 -> RO1/1
  single2/1 -> RO1/2

== system lib_all/SubSystem660/SubSystem665 ==
  note: ***編集禁止***
  In1 : Inport
  In2 : Inport  Port=2
  RO1 : RelationalOperator  Operator===  InputSameDT=on
  single1 : DataTypeConversion  OutDataTypeStr=single  SaturateOnIntegerOverflow=on  RndMeth=Zero
  single2 : DataTypeConversion  OutDataTypeStr=single  SaturateOnIntegerOverflow=on  RndMeth=Zero
  Out1 : Outport
  wires:
  RO1/1 -> Out1/1
  In2/1 -> single2/1
  In1/1 -> single1/1
  single1/1 -> RO1/1
  single2/1 -> RO1/2

== system lib_all/SubSystem660/SubSystem666 ==
  note: ***編集禁止***
  In1 : Inport
  In2 : Inport  Port=2
  RO1 : RelationalOperator  Operator===  InputSameDT=on
  single1 : DataTypeConversion  OutDataTypeStr=single  SaturateOnIntegerOverflow=on  RndMeth=Zero
  single2 : DataTypeConversion  OutDataTypeStr=single  SaturateOnIntegerOverflow=on  RndMeth=Zero
  Out1 : Outport
  wires:
  RO1/1 -> Out1/1
  In2/1 -> single2/1
  In1/1 -> single1/1
  single1/1 -> RO1/1
  single2/1 -> RO1/2

== system lib_all/SubSystem660/SubSystem667 ==
  note: ***編集禁止***
  In1 : Inport
  In2 : Inport  Port=2
  RO1 : RelationalOperator  Operator===  InputSameDT=on
  single1 : DataTypeConversion  OutDataTypeStr=single  SaturateOnIntegerOverflow=on  RndMeth=Zero
  single2 : DataTypeConversion  OutDataTypeStr=single  SaturateOnIntegerOverflow=on  RndMeth=Zero
  Out1 : Outport
  wires:
  RO1/1 -> Out1/1
  In2/1 -> single2/1
  In1/1 -> single1/1
  single1/1 -> RO1/1
  single2/1 -> RO1/2

== system lib_all/SubSystem660/SubSystem668 ==
  Block1483 : Inport
  Block1484 : Inport  Port=2
  Block1485 : EnablePort
  Block1486 : DataStoreWrite  DataStoreName=DSM237
  Block1487 : DataStoreWrite  DataStoreName=DSM232
  wires:
  Block1484/1 -> Block1487/1
  Block1483/1 -> Block1486/1

== system lib_all/SubSystem660/SubSystem669 ==
  Block1488 : Inport
  Block1489 : Inport  Port=2
  Block1490 : EnablePort
  Block1491 : DataStoreWrite  DataStoreName=DSM238
  Block1492 : DataStoreWrite  DataStoreName=DSM233
  wires:
  Block1489/1 -> Block1492/1
  Block1488/1 -> Block1491/1

== system lib_all/SubSystem660/SubSystem670 ==
  Block1493 : Inport
  Block1494 : Inport  Port=2
  Block1495 : EnablePort
  Block1496 : DataStoreWrite  DataStoreName=DSM239
  Block1497 : DataStoreWrite  DataStoreName=DSM234
  wires:
  Block1494/1 -> Block1497/1
  Block1493/1 -> Block1496/1

== system lib_all/SubSystem660/SubSystem671 ==
  Block1498 : Inport
  Block1499 : Inport  Port=2
  Block1500 : EnablePort
  Block1501 : DataStoreWrite  DataStoreName=DSM240
  Block1502 : DataStoreWrite  DataStoreName=DSM235
  wires:
  Block1499/1 -> Block1502/1
  Block1498/1 -> Block1501/1

== system lib_all/SubSystem660/SubSystem672 ==
  Block1503 : Inport
  Block1504 : Inport  Port=2
  Block1505 : EnablePort
  Block1506 : DataStoreWrite  DataStoreName=DSM242
  Block1507 : DataStoreWrite  DataStoreName=DSM236
  wires:
  Block1504/1 -> Block1507/1
  Block1503/1 -> Block1506/1

== system lib_all/SubSystem660/SubSystem673 ==
  note: Text97
  note: Text96
  Block1508 : Inport
  Block1509 : Inport  Port=2
  Block1510 : Inport  Port=3
  Block1511 : Constant  Value=0  OutDataTypeStr=double  SampleTime=-1
  SubSystem675 : Subsystem
  SubSystem676 : Subsystem
  SubSystem683 : Subsystem  TreatAsAtomicUnit=on
  SubSystem684 : Subsystem
  Block1553 : Outport
  wires:
  Block1509/1 -> SubSystem676/1(Block1516)
  Block1508/1 -> SubSystem676/2(Block1517), [TagName4]
  SubSystem684/1(Block1552) -> Block1553/1, [TagName24]
  SubSystem676/1(Block1541) -> SubSystem684/2(Block1546)
  Block1510/1 -> SubSystem684/1(Block1545)
  [TagName4] -> SubSystem675/1(In1)
  Block1511/1 -> SubSystem675/2(In2)
  SubSystem675/1(Out1) -> SubSystem683/Enable
  [TagName24] -> SubSystem683/1(Block1542)

== system lib_all/SubSystem660/SubSystem686 ==
  note: Text105
  note: Text104
  note: Text103
  Block1554 : Inport
  Block1555 : Inport  Port=2
  Block1556 : Inport  Port=3
  Block1557 : Constant  OutDataTypeStr=double  SampleTime=-1
  Block1558 : Gain  Gain=1/5  SaturateOnIntegerOverflow=on  OutDataTypeStr=Inherit: Same as input  ParamDataTypeStr=Inherit: Same as input
      settings: AttributesFormatString=Attribute328
  Block1559 : Product  OutDataTypeStr=double  SaturateOnIntegerOverflow=on  RndMeth=Zero  InputSameDT=on
  Block1560 : Sum  Inputs=-+  OutDataTypeStr=double  RndMeth=Zero  SaturateOnIntegerOverflow=on  InputSameDT=on
  Block1561 : Sum  OutDataTypeStr=double  RndMeth=Zero  SaturateOnIntegerOverflow=on  InputSameDT=on
  Block1562 : Sum  OutDataTypeStr=double  RndMeth=Zero  SaturateOnIntegerOverflow=on  InputSameDT=on
  SubSystem688 : Subsystem
  SubSystem694 : Subsystem
  Block1653 : Outport
  wires:
  Block1562/1 -> SubSystem694/3(Block1591), SubSystem688/2(Block1564)
  Block1554/1 -> Block1562/1
  Block1558/1 -> Block1561/2
  Block1559/1 -> Block1558/1
  Block1557/1 -> Block1562/2
  Block1561/1 -> Block1653/1
  SubSystem688/2(Block1588) -> Block1559/2
  Block1560/1 -> Block1559/1  "SigLabel40"
  SubSystem694/2(Block1652) -> Block1560/2
  SubSystem694/1(Block1651) -> Block1560/1, Block1561/1
  Block1556/1 -> SubSystem694/2(Block1590)
  SubSystem688/1(Block1587) -> SubSystem694/1(Block1589)
  Block1555/1 -> SubSystem688/1(Block1563)

== system lib_all/SubSystem7/SubSystem134 ==
  note: Text22
  Block311 : Inport
  Block312 : Constant  Value=Parameter18  OutDataTypeStr=double  SampleTime=-1
  Block313 : Constant  OutDataTypeStr=double  SampleTime=-1
  Block314 : Constant  OutDataTypeStr=double  SampleTime=-1
  Block315 : Sum  OutDataTypeStr=double  RndMeth=Zero  SaturateOnIntegerOverflow=on  InputSameDT=on
  Block316 : Sum  OutDataTypeStr=double  RndMeth=Zero  SaturateOnIntegerOverflow=on  InputSameDT=on
  SubSystem135 : Subsystem
  SubSystem136 : Subsystem
  SubSystem159 : Subsystem  TreatAsAtomicUnit=on  mask(***ATC.DSRVEC***){bd_version=1.01.A, r0=DSM374, r1=-1, r2=, r3=, r4=, r5=, r6=, r7=, r8=, r9=, c0=, c1=, c2=, c3=, c4=, c5=, c6=, c7=, c8=, c9=}
      mask prompts: bd_version "Version"
      mask help: これはAutoCからのブロックです．編集禁止．
      settings: OpenFcn=warndlg('変更禁止!');
      mask Help: web(ui_blockhelpmng(gcb));
      mask Display: disp('ATC.DSRVEC')
  SubSystem160 : Subsystem  TreatAsAtomicUnit=on  mask(***ATC.DSWVEC***){bd_version=1.01.A, r0=DSM374, r1=-1, r2=, r3=, r4=, r5=, r6=, r7=, r8=, r9=, c0=, c1=, c2=, c3=, c4=, c5=, c6=, c7=, c8=, c9=}
      mask prompts: bd_version "Version"
      mask help: これはAutoCからのブロックです．編集禁止．
      settings: OpenFcn=warndlg('変更禁止!');
      mask Help: web(ui_blockhelpmng(gcb));
      mask Display: disp('ATC.DSRVEC')
  wires:
  Block316/1 -> SubSystem160/1(input), SubSystem135/1(In1)
  Block315/1 -> SubSystem160/2(element), SubSystem159/1(element), SubSystem136/1(Block317)
  Block311/1 -> Block315/1
  Block312/1 -> SubSystem135/2(In2)
  SubSystem135/1(Out1) -> SubSystem136/Enable
  Block313/1 -> Block315/2
  SubSystem159/1(Output) -> Block316/1
  Block314/1 -> Block316/2

== system lib_all/SubSystem7/SubSystem161 ==
  note: Text26
  Block363 : Constant  Value=Parameter18  OutDataTypeStr=double  SampleTime=-1
  Block364 : Constant  Value=Parameter131  OutDataTypeStr=double  SampleTime=-1
  Block365 : DataStoreRead  DataStoreName=DSM374
  Block366 : Sum  Inputs=+  OutDataTypeStr=double  RndMeth=Zero  SaturateOnIntegerOverflow=on  InputSameDT=on
  SubSystem162 : Subsystem
  SubSystem163 : Subsystem
  SubSystem164 : Subsystem  TreatAsAtomicUnit=on
  wires:
  Block365/1 -> SubSystem162/1(In1)
  Block363/1 -> SubSystem162/2(In2)
  SubSystem162/1(Out1) -> Block366/1
  Block366/1 -> SubSystem163/1(In1)
  Block364/1 -> SubSystem163/2(In2)
  SubSystem163/1(Out1) -> SubSystem164/Enable

== system lib_all/SubSystem7/SubSystem9 ==
  note: Text18
  Block11 : Inport
  Block12 : Constant  Value=Parameter18  OutDataTypeStr=double  SampleTime=-1
  Block13 : Constant  OutDataTypeStr=double  SampleTime=-1
  Block14 : Demux  Outputs=2
  Block15 : Sum  OutDataTypeStr=double  RndMeth=Zero  SaturateOnIntegerOverflow=on  InputSameDT=on
  SubSystem10 : Subsystem
  SubSystem11 : Subsystem
      settings: RTWSystemCode=Function  RTWFcnNameOpts=Use subsystem name
  SubSystem12 : Subsystem  TreatAsAtomicUnit=on  mask(***ATC.DSRVEC***){bd_version=1.01.A, r0=DSM374, r1=-1, r2=, r3=, r4=, r5=, r6=, r7=, r8=, r9=, c0=, c1=, c2=, c3=, c4=, c5=, c6=, c7=, c8=, c9=}
      mask prompts: bd_version "Version"
      mask help: これはAutoCからのブロックです．編集禁止．
      settings: OpenFcn=warndlg('変更禁止!');
      mask Help: web(ui_blockhelpmng(gcb));
      mask Display: disp('ATC.DSRVEC')
  SubSystem13 : Subsystem
  wires:
  Block15/1 -> SubSystem12/1(element), SubSystem11/1(Block16)
  SubSystem11/1(Block27) -> Block14/1
  Block14/1 -> SubSystem13/2(Block29)  "SigLabel8"
  Block14/2 -> SubSystem13/3(Block30)  "SigLabel7"
  Block11/1 -> Block15/1, SubSystem13/1(Block28)
  SubSystem12/1(Output) -> SubSystem10/1(In1), SubSystem13/4(Block31)
  Block12/1 -> SubSystem10/2(In2)
  SubSystem10/1(Out1) -> SubSystem13/Enable
  Block13/1 -> Block15/2

== system lib_all/SubSystem727/SubSystem729 ==
  note: ***編集禁止***
  In1 : Inport
  In2 : Inport  Port=2
  RO1 : RelationalOperator  Operator===  InputSameDT=on
  single1 : DataTypeConversion  OutDataTypeStr=single  SaturateOnIntegerOverflow=on  RndMeth=Zero
  single2 : DataTypeConversion  OutDataTypeStr=single  SaturateOnIntegerOverflow=on  RndMeth=Zero
  Out1 : Outport
  wires:
  single2/1 -> RO1/2
  single1/1 -> RO1/1
  In1/1 -> single1/1
  In2/1 -> single2/1
  RO1/1 -> Out1/1

== system lib_all/SubSystem727/SubSystem730 ==
  note: ***編集禁止***
  In1 : Inport
  In2 : Inport  Port=2
  RO1 : RelationalOperator  Operator===  InputSameDT=on
  single1 : DataTypeConversion  OutDataTypeStr=single  SaturateOnIntegerOverflow=on  RndMeth=Zero
  single2 : DataTypeConversion  OutDataTypeStr=single  SaturateOnIntegerOverflow=on  RndMeth=Zero
  Out1 : Outport
  wires:
  single2/1 -> RO1/2
  single1/1 -> RO1/1
  In1/1 -> single1/1
  In2/1 -> single2/1
  RO1/1 -> Out1/1

== system lib_all/SubSystem727/SubSystem731 ==
  note: ***編集禁止***
  In1 : Inport
  In2 : Inport  Port=2
  RO1 : RelationalOperator  Operator===  InputSameDT=on
  single1 : DataTypeConversion  OutDataTypeStr=single  SaturateOnIntegerOverflow=on  RndMeth=Zero
  single2 : DataTypeConversion  OutDataTypeStr=single  SaturateOnIntegerOverflow=on  RndMeth=Zero
  Out1 : Outport
  wires:
  single2/1 -> RO1/2
  single1/1 -> RO1/1
  In1/1 -> single1/1
  In2/1 -> single2/1
  RO1/1 -> Out1/1

== system lib_all/SubSystem727/SubSystem732 ==
  note: ***編集禁止***
  In1 : Inport
  In2 : Inport  Port=2
  RO1 : RelationalOperator  Operator===  InputSameDT=on
  single1 : DataTypeConversion  OutDataTypeStr=single  SaturateOnIntegerOverflow=on  RndMeth=Zero
  single2 : DataTypeConversion  OutDataTypeStr=single  SaturateOnIntegerOverflow=on  RndMeth=Zero
  Out1 : Outport
  wires:
  single2/1 -> RO1/2
  single1/1 -> RO1/1
  In1/1 -> single1/1
  In2/1 -> single2/1
  RO1/1 -> Out1/1

== system lib_all/SubSystem727/SubSystem733 ==
  note: ***編集禁止***
  In1 : Inport
  In2 : Inport  Port=2
  RO1 : RelationalOperator  Operator===  InputSameDT=on
  single1 : DataTypeConversion  OutDataTypeStr=single  SaturateOnIntegerOverflow=on  RndMeth=Zero
  single2 : DataTypeConversion  OutDataTypeStr=single  SaturateOnIntegerOverflow=on  RndMeth=Zero
  Out1 : Outport
  wires:
  single2/1 -> RO1/2
  single1/1 -> RO1/1
  In1/1 -> single1/1
  In2/1 -> single2/1
  RO1/1 -> Out1/1

== system lib_all/SubSystem727/SubSystem734 ==
  note: ***編集禁止***
  In1 : Inport
  In2 : Inport  Port=2
  RO1 : RelationalOperator  Operator===  InputSameDT=on
  single1 : DataTypeConversion  OutDataTypeStr=single  SaturateOnIntegerOverflow=on  RndMeth=Zero
  single2 : DataTypeConversion  OutDataTypeStr=single  SaturateOnIntegerOverflow=on  RndMeth=Zero
  Out1 : Outport
  wires:
  single2/1 -> RO1/2
  single1/1 -> RO1/1
  In1/1 -> single1/1
  In2/1 -> single2/1
  RO1/1 -> Out1/1

== system lib_all/SubSystem727/SubSystem735 ==
  Block1685 : Inport
  Block1686 : Inport  Port=2
  Block1687 : Inport  Port=3
  Block1688 : EnablePort
  Block1689 : DataStoreWrite  DataStoreName=DSM20
  Block1690 : DataStoreWrite  DataStoreName=DSM30
  Block1691 : DataStoreWrite  DataStoreName=DSM25
  wires:
  Block1685/1 -> Block1689/1
  Block1686/1 -> Block1690/1
  Block1687/1 -> Block1691/1

== system lib_all/SubSystem727/SubSystem736 ==
  Block1692 : Inport
  Block1693 : Inport  Port=2
  Block1694 : Inport  Port=3
  Block1695 : EnablePort
  Block1696 : DataStoreWrite  DataStoreName=DSM21
  Block1697 : DataStoreWrite  DataStoreName=DSM31
  Block1698 : DataStoreWrite  DataStoreName=DSM26
  wires:
  Block1692/1 -> Block1696/1
  Block1693/1 -> Block1697/1
  Block1694/1 -> Block1698/1
